simulink model "CCMLin2Mgr"  (R2022b)
settings: Solver=FixedStepDiscrete  StartTime=0.0  StopTime=10.0  FixedStep=0.02  RelTol=1e-3
undefined symbols (not defined in the file or in its folder): ComChlType, NetworkChlType, P_LinIfSchHandleType, P_Std_ReturnType
(part 2 of 3)

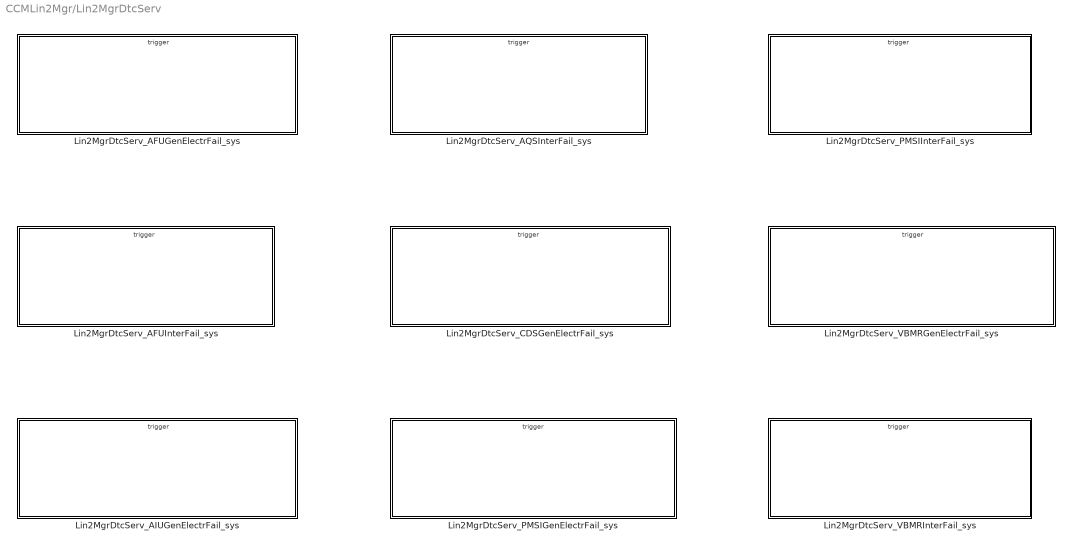
== system CCMLin2Mgr/Lin2MgrDtcServ ==
  Lin2MgrDtcServ_AFUGenElectrFail_sys : Subsystem
  Lin2MgrDtcServ_AFUInterFail_sys : Subsystem
  Lin2MgrDtcServ_AIUGenElectrFail_sys : Subsystem
  Lin2MgrDtcServ_AQSInterFail_sys : Subsystem
  Lin2MgrDtcServ_CDSGenElectrFail_sys : Subsystem
  Lin2MgrDtcServ_PMSIGenElectrFail_sys : Subsystem
  Lin2MgrDtcServ_PMSIInterFail_sys : Subsystem
  Lin2MgrDtcServ_VBMRGenElectrFail_sys : Subsystem
  Lin2MgrDtcServ_VBMRInterFail_sys : Subsystem

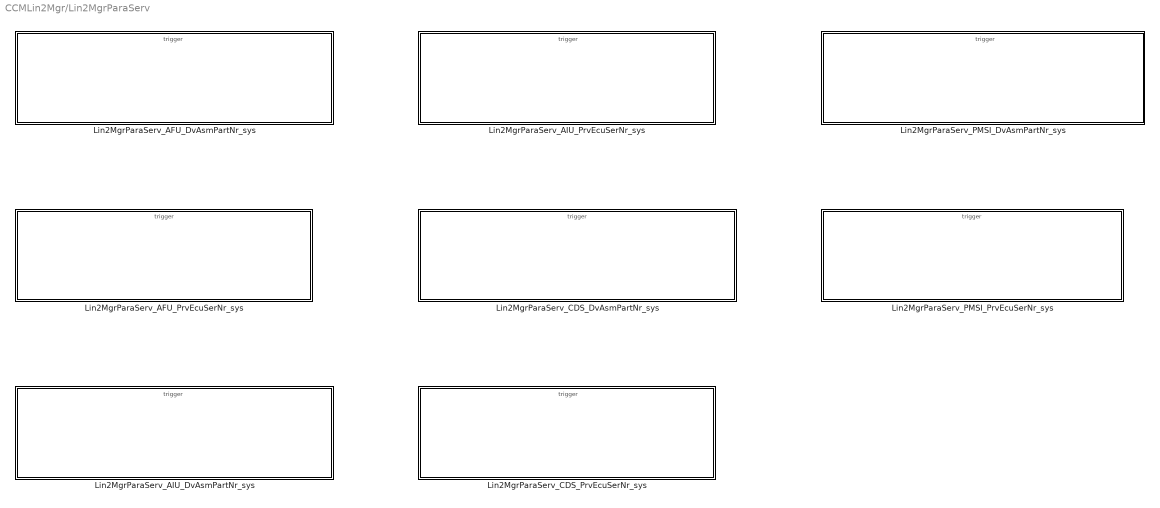
== system CCMLin2Mgr/Lin2MgrParaServ ==
  Lin2MgrParaServ_AFU_DvAsmPartNr_sys : Subsystem
  Lin2MgrParaServ_AFU_PrvEcuSerNr_sys : Subsystem
  Lin2MgrParaServ_AIU_DvAsmPartNr_sys : Subsystem
  Lin2MgrParaServ_AIU_PrvEcuSerNr_sys : Subsystem
  Lin2MgrParaServ_CDS_DvAsmPartNr_sys : Subsystem
  Lin2MgrParaServ_CDS_PrvEcuSerNr_sys : Subsystem
  Lin2MgrParaServ_PMSI_DvAsmPartNr_sys : Subsystem
  Lin2MgrParaServ_PMSI_PrvEcuSerNr_sys : Subsystem

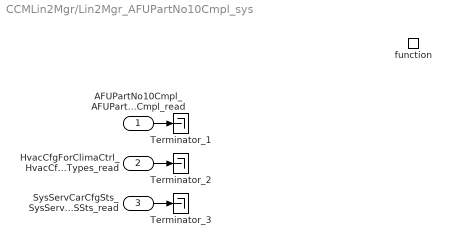
== system CCMLin2Mgr/Lin2Mgr_AFUPartNo10Cmpl_sys ==
  AFUPartNo10Cmpl_AFUPartNo10Cmpl_read : Inport
  HvacCfgForClimaCtrl_HvacCfgForAQSTypes_read : Inport  Port=2
  SysServCarCfgSts_SysServCCPAQSSts_read : Inport  Port=3
  function : TriggerPort  TriggerType=function-call  VariantControl=(inherit)
  Terminator_1 : Terminator
  Terminator_2 : Terminator
  Terminator_3 : Terminator
  wires:
  AFUPartNo10Cmpl_AFUPartNo10Cmpl_read/1 -> Terminator_1/1
  HvacCfgForClimaCtrl_HvacCfgForAQSTypes_read/1 -> Terminator_2/1
  SysServCarCfgSts_SysServCCPAQSSts_read/1 -> Terminator_3/1

== system CCMLin2Mgr/Lin2Mgr_AFUPartNoCmpl_sys ==
  AFUPartNoCmpl_AFUPartNoCmpl_read : Inport
  function : TriggerPort  TriggerType=function-call  VariantControl=(inherit)
  Terminator_1 : Terminator
  wires:
  AFUPartNoCmpl_AFUPartNoCmpl_read/1 -> Terminator_1/1

== system CCMLin2Mgr/Lin2Mgr_AFUSerNo_sys ==
  AFUSerNo_AFUSerNo_read : Inport
  function : TriggerPort  TriggerType=function-call  VariantControl=(inherit)
  Terminator_1 : Terminator
  wires:
  AFUSerNo_AFUSerNo_read/1 -> Terminator_1/1

== system CCMLin2Mgr/Lin2Mgr_AIUPartNo10Cmpl_sys ==
  AIUPartNo10Cmpl_AIUPartNo10Cmpl_read : Inport
  function : TriggerPort  TriggerType=function-call  VariantControl=(inherit)
  Terminator_1 : Terminator
  wires:
  AIUPartNo10Cmpl_AIUPartNo10Cmpl_read/1 -> Terminator_1/1

== system CCMLin2Mgr/Lin2Mgr_AIUPartNoCmpl_sys ==
  AIUPartNoCmpl_AIUPartNoCmpl_read : Inport
  function : TriggerPort  TriggerType=function-call  VariantControl=(inherit)
  Terminator_1 : Terminator
  wires:
  AIUPartNoCmpl_AIUPartNoCmpl_read/1 -> Terminator_1/1

== system CCMLin2Mgr/Lin2Mgr_AIUSerNo_sys ==
  AIUSerNo_AIUSerNo_read : Inport
  function : TriggerPort  TriggerType=function-call  VariantControl=(inherit)
  Terminator_1 : Terminator
  wires:
  AIUSerNo_AIUSerNo_read/1 -> Terminator_1/1

== system CCMLin2Mgr/Lin2Mgr_CDSPartNo10Cmpl_sys ==
  CDSPartNo10Cmpl_CDSPartNo10Cmpl_read : Inport
  function : TriggerPort  TriggerType=function-call  VariantControl=(inherit)
  Terminator_1 : Terminator
  wires:
  CDSPartNo10Cmpl_CDSPartNo10Cmpl_read/1 -> Terminator_1/1

== system CCMLin2Mgr/Lin2Mgr_CDSPartNoCmpl_sys ==
  CDSPartNoCmpl_CDSPartNoCmpl_read : Inport
  function : TriggerPort  TriggerType=function-call  VariantControl=(inherit)
  Terminator_1 : Terminator
  wires:
  CDSPartNoCmpl_CDSPartNoCmpl_read/1 -> Terminator_1/1

== system CCMLin2Mgr/Lin2Mgr_CDSSerNo_sys ==
  CDSSerNo_CDSSerNo_read : Inport
  function : TriggerPort  TriggerType=function-call  VariantControl=(inherit)
  Terminator_1 : Terminator
  wires:
  CDSSerNo_CDSSerNo_read/1 -> Terminator_1/1

== system CCMLin2Mgr/Lin2Mgr_Init ==
  Event Listener : EventListener  EventName=reset

== system CCMLin2Mgr/Lin2Mgr_KeepComActv_sys ==
  KeepComActvL2_KeepComActvL2_read : Inport
  function : TriggerPort  TriggerType=function-call  VariantControl=(inherit)  SampleTimeType=periodic  SampleTime=-1
  Terminator_1 : Terminator
  wires:
  KeepComActvL2_KeepComActvL2_read/1 -> Terminator_1/1

== system CCMLin2Mgr/Lin2Mgr_PMSIPartNo10Hw_sys ==
  PMSIPartNo10Hw_PMSIPartNo10Hw_read : Inport
  function : TriggerPort  TriggerType=function-call  VariantControl=(inherit)
  Terminator_1 : Terminator
  wires:
  PMSIPartNo10Hw_PMSIPartNo10Hw_read/1 -> Terminator_1/1

== system CCMLin2Mgr/Lin2Mgr_PMSIPartNoHw_sys ==
  PMSIPartNoHw_PMSIPartNoHw_read : Inport
  function : TriggerPort  TriggerType=function-call  VariantControl=(inherit)
  Terminator_1 : Terminator
  wires:
  PMSIPartNoHw_PMSIPartNoHw_read/1 -> Terminator_1/1

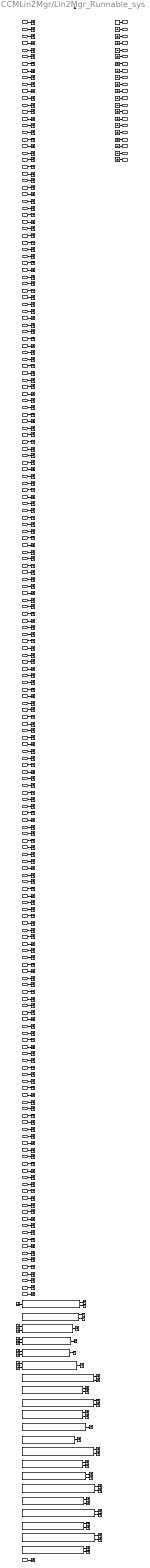
== system CCMLin2Mgr/Lin2Mgr_Runnable_sys ==
  RlyCmdCmft_RlyCmdCmft_read : Inport
  SysServCarCfgSts_SysServCarID_read : Inport  Port=2
  SysServCarCfgSts_SysServCCPAQSSts_read : Inport  Port=3
  SysServCarCfgSts_SysServCCPHEXSensorSts_read : Inport  Port=4
  SysServCarCfgSts_SysServCCPLin2AFUSts_read : Inport  Port=5
  SysServCarCfgSts_SysServCCPLin2AIUSts_read : Inport  Port=6
  SysServCarCfgSts_SysServCCPLin2CDSSts_read : Inport  Port=7
  SysServCarCfgSts_SysServCCPLin2PMSISts_read : Inport  Port=8
  SysServCarCfgSts_SysServCCPLin3VGASts_read : Inport  Port=9
  SysServCarCfgSts_SysServDUAirVentSnrSts_read : Inport  Port=10
  SysServCarCfgSts_SysServDUBlowerPwmSignSts_read : Inport  Port=11
  SysServCarCfgSts_SysServDUBlowerPwmSignTypeSts_read : Inport  Port=12
  SysServCarCfgSts_SysServDUBlowerPwmSts_read : Inport  Port=13
  SysServCarCfgSts_SysServDUDCDefrostSts_read : Inport  Port=14
  SysServCarCfgSts_SysServDUDCModeSts_read : Inport  Port=15
  SysServCarCfgSts_SysServDUDCRecSts_read : Inport  Port=16
  SysServCarCfgSts_SysServDUDCSModeSts_read : Inport  Port=17
  SysServCarCfgSts_SysServDUDCSTLSts_read : Inport  Port=18
  SysServCarCfgSts_SysServDUDCTLSts_read : Inport  Port=19
  SysServCarCfgSts_SysServDUDCTRSts_read : Inport  Port=20
  SysServCarCfgSts_SysServDUFLFootSts_read : Inport  Port=21
  SysServCarCfgSts_SysServDUFLVentSts_read : Inport  Port=22
  SysServCarCfgSts_SysServDUFRFootSts_read : Inport  Port=23
  SysServCarCfgSts_SysServDUFRVentSts_read : Inport  Port=24
  SysServCarCfgSts_SysServDUIncarMotorSts_read : Inport  Port=25
  SysServCarCfgSts_SysServDULin1HBMFSts_read : Inport  Port=26
  SysServCarCfgSts_SysServDULin4HFAFSts_read : Inport  Port=27
  SysServCarCfgSts_SysServDUSFLFootSts_read : Inport  Port=28
  SysServCarCfgSts_SysServDUSFLVentSts_read : Inport  Port=29
  SysServCarCfgSts_SysServDUSFRFootSts_read : Inport  Port=30
  SysServCarCfgSts_SysServDUSFRVentSts_read : Inport  Port=31
  SysServCarCfgSts_SysServDUSnsrForFCmptmtTSts_read : Inport  Port=32
  SysServCarCfgSts_SysServDUSnsrForRair_read : Inport  Port=33
  SysServCarCfgSts_SysServDUSnsrForRCmptmtTSts_read : Inport  Port=34
  AACAirClngErrSts_AACAirClngErrSts_read : Inport  Port=35
  AFUGenErr_AFUGenErr_read : Inport  Port=36
  AFUPartNo10Cmpl_AFUPartNo10Cmpl_read : Inport  Port=37
  AFUPartNoCmpl_AFUPartNoCmpl_read : Inport  Port=38
  AFUSerNo_AFUSerNo_read : Inport  Port=39
  AirClngErrSts_AirClngErrSts_read : Inport  Port=40
  AirFragFanErr_AirFragFanErr_read : Inport  Port=41
  AirFragMot1Err_AirFragMot1Err_read : Inport  Port=42
  AirFragMot2Err_AirFragMot2Err_read : Inport  Port=43
  AirFragMot3Err_AirFragMot3Err_read : Inport  Port=44
  AirFragMot4Err_AirFragMot4Err_read : Inport  Port=45
  AirFragMot5Err_AirFragMot5Err_read : Inport  Port=46
  AirFragOvrVoltErr_AirFragOvrVoltErr_read : Inport  Port=47
  AirFragTempErr_AirFragTempErr_read : Inport  Port=48
  AirFragUndVoltErr_AirFragUndVoltErr_read : Inport  Port=49
  AIUPartNo10Cmpl_AIUPartNo10Cmpl_read : Inport  Port=50
  AIUPartNoCmpl_AIUPartNoCmpl_read : Inport  Port=51
  AIUSerNo_AIUSerNo_read : Inport  Port=52
  AQSAirQuality_AQSAirQuality_read : Inport  Port=53
  AQSCOxstatus_AQSCOxstatus_read : Inport  Port=54
  AQSNH3status_AQSNH3status_read : Inport  Port=55
  AQSNoxStatus_AQSNoxStatus_read : Inport  Port=56
  CarCfgForClimaCtrl_CarCfgForAirClng_read : Inport  Port=57
  CarCfgForClimaCtrl_CarCfgForAirClnrAdv_read : Inport  Port=58
  CarCfgForClimaCtrl_CarCfgForAirFrag_read : Inport  Port=59
  CarCfgForClimaCtrl_CarCfgForAirMtrlSnsr_read : Inport  Port=60
  CarCfgForClimaCtrl_CarCfgForAirQly_read : Inport  Port=61
  CarCfgForClimaCtrl_CarCfgForAmntOfSeat_read : Inport  Port=62
  CarCfgForClimaCtrl_CarCfgForAutDemst_read : Inport  Port=63
  CarCfgForClimaCtrl_CarCfgForAuxWtrPmp_read : Inport  Port=64
  CarCfgForClimaCtrl_CarCfgForBRAND_read : Inport  Port=65
  CarCfgForClimaCtrl_CarCfgForBypVlvInHvac_read : Inport  Port=66
  CarCfgForClimaCtrl_CarCfgForCabinOxgenSupply_read : Inport  Port=67
  CarCfgForClimaCtrl_CarCfgForClsVlvInHvac_read : Inport  Port=68
  CarCfgForClimaCtrl_CarCfgForCmprAcTyp_read : Inport  Port=69
  CarCfgForClimaCtrl_CarCfgForCmptmtClng_read : Inport  Port=70
  CarCfgForClimaCtrl_CarCfgForElectricLiftingModule_read : Inport  Port=71
  CarCfgForClimaCtrl_CarCfgForElecVentAdjmt_read : Inport  Port=72
  CarCfgForClimaCtrl_CarCfgForExtVentnClimaCtrl_read : Inport  Port=73
  CarCfgForClimaCtrl_CarCfgForFlcTyp_read : Inport  Port=74
  CarCfgForClimaCtrl_CarCfgForFoldCSD_read : Inport  Port=75
  CarCfgForClimaCtrl_CarCfgForFuTyp_read : Inport  Port=76
  CarCfgForClimaCtrl_CarCfgForGlassTyp_read : Inport  Port=77
  CarCfgForClimaCtrl_CarCfgForHeadRadar_read : Inport  Port=78
  CarCfgForClimaCtrl_CarCfgForHeatPmp_read : Inport  Port=79
  CarCfgForClimaCtrl_CarCfgForHeatrDurgDrvg_read : Inport  Port=80
  CarCfgForClimaCtrl_CarCfgForHeatrTyp_read : Inport  Port=81
  CarCfgForClimaCtrl_CarCfgForHvacFilTyp_read : Inport  Port=82
  CarCfgForClimaCtrl_CarCfgForHvacFlrSnsrForT_read : Inport  Port=83
  CarCfgForClimaCtrl_CarCfgForHvacHeatrSnsrT_read : Inport  Port=84
  CarCfgForClimaCtrl_CarCfgForHvacRe_read : Inport  Port=85
  CarCfgForClimaCtrl_CarCfgForHvacTyp_read : Inport  Port=86
  CarCfgForClimaCtrl_CarCfgForInteriorViewCamera_read : Inport  Port=87
  CarCfgForClimaCtrl_CarCfgForLifeDetection_read : Inport  Port=88
  CarCfgForClimaCtrl_CarCfgForLoVoltElecCfg_read : Inport  Port=89
  CarCfgForClimaCtrl_CarCfgForNavSysandIntnmap_read : Inport  Port=90
  CarCfgForClimaCtrl_CarCfgForNrOfDoor_read : Inport  Port=91
  CarCfgForClimaCtrl_CarCfgForPipeInFuTank_read : Inport  Port=92
  CarCfgForClimaCtrl_CarCfgForPowerSeats_read : Inport  Port=93
  CarCfgForClimaCtrl_CarCfgForPreClng_read : Inport  Port=94
  CarCfgForClimaCtrl_CarCfgForPrkgCDSNotAvl_read : Inport  Port=95
  CarCfgForClimaCtrl_CarCfgForPrkgClima_read : Inport  Port=96
  CarCfgForClimaCtrl_CarCfgForPrpsnTyp_read : Inport  Port=97
  CarCfgForClimaCtrl_CarCfgForPTCInFrontHVAC_read : Inport  Port=98
  CarCfgForClimaCtrl_CarCfgForRemEngStrtPreCdn_read : Inport  Port=99
  CarCfgForClimaCtrl_CarCfgForRoofHatch_read : Inport  Port=100
  CarCfgForClimaCtrl_CarCfgForSeatHeatgCal_read : Inport  Port=101
  CarCfgForClimaCtrl_CarCfgForSeatHeatgForRowFirst_read : Inport  Port=102
  CarCfgForClimaCtrl_CarCfgForSeatHeatgForRowSec_read : Inport  Port=103
  CarCfgForClimaCtrl_CarCfgForSeatVentnForRowFirst_read : Inport  Port=104
  CarCfgForClimaCtrl_CarCfgForSeatVentnForRowSec_read : Inport  Port=105
  CarCfgForClimaCtrl_CarCfgForSecRowVentnCenter_read : Inport  Port=106
  CarCfgForClimaCtrl_CarCfgForSecRowVentnSide_read : Inport  Port=107
  CarCfgForClimaCtrl_CarCfgForSmallFlowCyc_read : Inport  Port=108
  CarCfgForClimaCtrl_CarCfgForSmkSnsr_read : Inport  Port=109
  CarCfgForClimaCtrl_CarCfgForSnsrForCmptmtT_read : Inport  Port=110
  CarCfgForClimaCtrl_CarCfgForSnsrHumPrsnt_read : Inport  Port=111
  CarCfgForClimaCtrl_CarCfgForStartDevice_read : Inport  Port=112
  CarCfgForClimaCtrl_CarCfgForSteerWhlHeatg_read : Inport  Port=113
  CarCfgForClimaCtrl_CarCfgForSteerWhlPosn_read : Inport  Port=114
  CarCfgForClimaCtrl_CarCfgForSunSnsr_read : Inport  Port=115
  CarCfgForClimaCtrl_CarCfgForTelmNodTyp_read : Inport  Port=116
  CarCfgForClimaCtrl_CarCfgForThermMngt_read : Inport  Port=117
  CarCfgForClimaCtrl_CarCfgForVehTyp_read : Inport  Port=118
  CarCfgForClimaCtrl_CarCfgForVentLiftMotorExiSts_read : Inport  Port=119
  CarCfgForClimaCtrl_CarCfgForVgaMotorExistSts_read : Inport  Port=120
  CarCfgForClimaCtrl_CarCfgForWadingAssistSystem_read : Inport  Port=121
  CarCfgForClimaCtrl_CarCfgForWindHeatd_read : Inport  Port=122
  CarCfgForClimaCtrl_CarCfgWindFollowHumanMov_read : Inport  Port=123
  CDSPartNo10Cmpl_CDSPartNo10Cmpl_read : Inport  Port=124
  CDSPartNoCmpl_CDSPartNoCmpl_read : Inport  Port=125
  CDSSerNo_CDSSerNo_read : Inport  Port=126
  ClimRlyCmd_ClimRlyCmd_read : Inport  Port=127
  CmpmtInAirPmAQI_CmpmtInAirPmAQI_read : Inport  Port=128
  CmpmtInAirPmDnsty_CmpmtInAirPmDnsty_read : Inport  Port=129
  CmptmtOxygenConcQFNgcc_CmptmtOxygenConcQFNgcc_read : Inport  Port=130
  CmptmtOxygenConcVluNgcc_CmptmtOxygenConcVluNgcc_read : Inport  Port=131
  HvacCfgForClimaCtrl_HvacCfgForAQSTypes_read : Inport  Port=132
  HvacCfgForClimaCtrl_HvacCfgForHvacFrontBlowerType_read : Inport  Port=133
  HvacCfgForClimaCtrl_HvacCfgForHvacLowSideRETXVESts_read : Inport  Port=134
  HvacCfgForClimaCtrl_HvacCfgForHvacMiscExistSts_read : Inport  Port=135
  HvacCfgForClimaCtrl_HvacCfgForHvacMotorExistSts_read : Inport  Port=136
  HvacCfgForClimaCtrl_HvacCfgForHvacReBlowerType_read : Inport  Port=137
  HvacCfgForClimaCtrl_HvacCfgForHvacReVentPstn_read : Inport  Port=138
  HvacCfgForClimaCtrl_HvacCfgForHvacSensorExistSts_read : Inport  Port=139
  HvacCfgForClimaCtrl_HvacCfgForHvacSnsrRCmptmtESts_read : Inport  Port=140
  HvacCfgForClimaCtrl_HvacCfgForHvacSnsrReHexESts_read : Inport  Port=141
  HvacFanReqRe_HvacFanReqRe_read : Inport  Port=142
  HvacFanSts2HvacFanBattU2_HvacFanSts2HvacFanBattU2_read : Inport  Port=143
  HvacFanSts2HvacFanExt2_HvacFanSts2HvacFanExt2_read : Inport  Port=144
  HvacFanSts2HvacFanHw2_HvacFanSts2HvacFanHw2_read : Inport  Port=145
  HvacFanSts2HvacFanMotU2_HvacFanSts2HvacFanMotU2_read : Inport  Port=146
  HvacFanSts2HvacFanReSts1_HvacFanSts2HvacFanReSts1_read : Inport  Port=147
  HvacFanSts2HvacFanReSts2_HvacFanSts2HvacFanReSts2_read : Inport  Port=148
  HvacFanSts2HvacFanSw2_HvacFanSts2HvacFanSw2_read : Inport  Port=149
  HvacFanSts2HvacFanT2_HvacFanSts2HvacFanT2_read : Inport  Port=150
  HvacFanVoltReqRe_HvacFanVoltReqRe_read : Inport  Port=151
  InPm25FanErr_InPm25FanErr_read : Inport  Port=152
  InPm25SensrIntErr_InPm25SensrIntErr_read : Inport  Port=153
  InPm25SnrTempErr_InPm25SnrTempErr_read : Inport  Port=154
  InPm25SnrVoltgRangErr_InPm25SnrVoltgRangErr_read : Inport  Port=155
  InPm25SnsrElecErr_InPm25SnsrElecErr_read : Inport  Port=156
  InPm25SnsrSts_InPm25SnsrSts_read : Inport  Port=157
  IntCo2CircElecErr_IntCo2CircElecErr_read : Inport  Port=158
  IntCo2HvErr_IntCo2HvErr_read : Inport  Port=159
  IntCo2LvErr_IntCo2LvErr_read : Inport  Port=160
  KeepComActvL2_KeepComActvL2_read : Inport  Port=161
  OxygenConcSnsrElecErr_OxygenConcSnsrElecErr_read : Inport  Port=162
  OxygenConcSnsrElecErrNgcc_OxygenConcSnsrElecErrNgcc_read : Inport  Port=163
  OxygenConcSnsrIntErr_OxygenConcSnsrIntErr_read : Inport  Port=164
  OxygenConcSnsrIntErrNgcc_OxygenConcSnsrIntErrNgcc_read : Inport  Port=165
  OxygenConcSnsrOvrVoltRangErr_OxygenConcSnsrOvrVoltRangErr_read : Inport  Port=166
  OxygenConcSnsrStsNgcc_OxygenConcSnsrStsNgcc_read : Inport  Port=167
  OxygenConcSnsrTempErr_OxygenConcSnsrTempErr_read : Inport  Port=168
  OxygenConcSnsrTempErrNgcc_OxygenConcSnsrTempErrNgcc_read : Inport  Port=169
  OxygenConcSnsrUndVoltRangErr_OxygenConcSnsrUndVoltRangErr_read : Inport  Port=170
  OxygenConcSnsrUndVoltRangErrNgcc_OxygenConcSnsrUndVoltRangErrNgcc_read : Inport  Port=171
  PMSIPartNo10Hw_PMSIPartNo10Hw_read : Inport  Port=172
  PMSIPartNoHw_PMSIPartNoHw_read : Inport  Port=173
  PwrSplyToSnsrForAirQly_PwrSplyToSnsrForAirQly_read : Inport  Port=174
  RHFAScheTableReq_Val_read : Inport  Port=175
  SerNoPMSI_SerNoPMSI_read : Inport  Port=176
  SysServCarCfgSts_SysServCCPBGMNum_read : Inport  Port=177
  SysServCarCfgSts_SysServCCPLin2AQSSts_read : Inport  Port=178
  SysServCarCfgSts_SysServDUDCFLMdeSts_read : Inport  Port=179
  SysServCarCfgSts_SysServDUReBlowerPwmSignSts_read : Inport  Port=180
  SysServCarCfgSts_SysServDUReBlowerPwmSts_read : Inport  Port=181
  SysServCarCfgSts_SysServFrntHEXSnsrPosn_read : Inport  Port=182
  SysServCarCfgSts_SysServLowSideRETXVSts_read : Inport  Port=183
  SysServCarCfgSts_SysServReHEXSnsrPosn_read : Inport  Port=184
  SysServCarCfgSts_SysServRHFASts_read : Inport  Port=185
  SysServCarCfgSts_SysServSnsrForFCmptmtTType_read : Inport  Port=186
  BswLifeCycle_LifeCycle_read : Inport  Port=187
  function : TriggerPort  TriggerType=function-call  VariantControl=(inherit)  SampleTimeType=periodic  SampleTime=-1
  Ground_{1..9,11..30} (29 alike) : Ground
  Ground_10 : Subsystem
  LinIfServ_GetScheduleInfo : FunctionCaller  FunctionPrototype=[ScheduleInfo,ERR] = LinIfServ_GetScheduleInfo(Channel)  InputArgumentSpecifications=NetworkChlType(0)  OutputArgumentSpecifications=P_LinIfScheduleInfoType, P_Std_ReturnType
  LinSMServ_ScheduleRequest : FunctionCaller  FunctionPrototype=ERR = LinSMServ_ScheduleRequest(network,schedule)  InputArgumentSpecifications=NetworkChlType(0), P_LinIfSchHandleType  OutputArgumentSpecifications=P_Std_ReturnType
  LinService_GetLin2WakeupStatus : FunctionCaller  FunctionPrototype=[WakSta,ERR] = LinService_GetLin2WakeupStatus()  OutputArgumentSpecifications=uint8(1), P_Std_ReturnType
  LinService_SendLin3dFrame : FunctionCaller  FunctionPrototype=ERR = LinService_SendLin3dFrame(channel,data)  InputArgumentSpecifications=ComChlType(0), uint8([1])  OutputArgumentSpecifications=P_Std_ReturnType
  LinService_SetLin3cFrame : FunctionCaller  FunctionPrototype=ERR = LinService_SetLin3cFrame(Channel,Data)  InputArgumentSpecifications=ComChlType(0), uint8([1])  OutputArgumentSpecifications=P_Std_ReturnType
  LinService_SetLinTable : FunctionCaller  FunctionPrototype=ERR = LinService_SetLinTable(Channel,Table)  InputArgumentSpecifications=ComChlType(0), uint8(1)  OutputArgumentSpecifications=P_Std_ReturnType
  MirrorLin2ErrServ_GetAQSCcmLin2Fr01NoResp : FunctionCaller  FunctionPrototype=ErrFlag = MirrorLin2ErrServ_GetAQSCcmLin2Fr01NoResp()  OutputArgumentSpecifications=uint8(1)
  MirrorLin2ErrServ_GetAQSRxErrSta : FunctionCaller  FunctionPrototype=ErrFlag = MirrorLin2ErrServ_GetAQSRxErrSta()  OutputArgumentSpecifications=uint8(1)
  MirrorLin2ErrServ_GetAfuCcmLin2Fr01NoResp : FunctionCaller  FunctionPrototype=[ErrFlag,ERR] = MirrorLin2ErrServ_GetAfuCcmLin2Fr01NoResp()  OutputArgumentSpecifications=uint8(1), P_Std_ReturnType
  MirrorLin2ErrServ_GetAfuRxErrSta : FunctionCaller  FunctionPrototype=[ErrFlag,ERR] = MirrorLin2ErrServ_GetAfuRxErrSta()  OutputArgumentSpecifications=uint8(1), P_Std_ReturnType
  MirrorLin2ErrServ_GetAiuCcmLin2Fr01NoResp : FunctionCaller  FunctionPrototype=[ErrFlag,ERR] = MirrorLin2ErrServ_GetAiuCcmLin2Fr01NoResp()  OutputArgumentSpecifications=uint8(1), P_Std_ReturnType
  MirrorLin2ErrServ_GetAiuRxErrSta : FunctionCaller  FunctionPrototype=[ErrFlag,ERR] = MirrorLin2ErrServ_GetAiuRxErrSta()  OutputArgumentSpecifications=uint8(1), P_Std_ReturnType
  MirrorLin2ErrServ_GetCdsCcmLin2Fr01NoResp : FunctionCaller  FunctionPrototype=[ErrFlag,ERR] = MirrorLin2ErrServ_GetCdsCcmLin2Fr01NoResp()  OutputArgumentSpecifications=uint8(1), P_Std_ReturnType
  MirrorLin2ErrServ_GetCdsRxErrSta : FunctionCaller  FunctionPrototype=[ErrFlag,ERR] = MirrorLin2ErrServ_GetCdsRxErrSta()  OutputArgumentSpecifications=uint8(1), P_Std_ReturnType
  MirrorLin2ErrServ_GetLinBusTxErrSta : FunctionCaller  FunctionPrototype=[ErrFlag,ERR] = MirrorLin2ErrServ_GetLinBusTxErrSta()  OutputArgumentSpecifications=uint8(1), P_Std_ReturnType
  MirrorLin2ErrServ_GetPmsiCcmLin2Fr01NoResp : FunctionCaller  FunctionPrototype=[ErrFlag,ERR] = MirrorLin2ErrServ_GetPmsiCcmLin2Fr01NoResp()  OutputArgumentSpecifications=uint8(1), P_Std_ReturnType
  MirrorLin2ErrServ_GetPmsiRxErrSta : FunctionCaller  FunctionPrototype=[ErrFlag,ERR] = MirrorLin2ErrServ_GetPmsiRxErrSta()  OutputArgumentSpecifications=uint8(1), P_Std_ReturnType
  MirrorLin2ErrServ_GetRhfaCcmLin2Fr01NoResp : FunctionCaller  FunctionPrototype=[ErrFlag,ERR] = MirrorLin2ErrServ_GetRhfaCcmLin2Fr01NoResp()  OutputArgumentSpecifications=uint8(1), P_Std_ReturnType
  MirrorLin2ErrServ_GetRhfaRxErrSta : FunctionCaller  FunctionPrototype=[ErrFlag,ERR] = MirrorLin2ErrServ_GetRhfaRxErrSta()  OutputArgumentSpecifications=uint8(1), P_Std_ReturnType
  MirrorLin2ErrServ_GetVbmrCcmLin2Fr01NoResp : FunctionCaller  FunctionPrototype=[ErrFlag,ERR] = MirrorLin2ErrServ_GetVbmrCcmLin2Fr01NoResp()  OutputArgumentSpecifications=uint8(1), P_Std_ReturnType
  MirrorLin2ErrServ_GetVbmrRxErrSta : FunctionCaller  FunctionPrototype=[ErrFlag,ERR] = MirrorLin2ErrServ_GetVbmrRxErrSta()  OutputArgumentSpecifications=uint8(1), P_Std_ReturnType
  Terminator_{1..223} (223 alike) : Terminator
  AirQlyLin_AirQlyLin_write : Outport
      settings: EnsureOutportIsVirtual=on
  HvacFanSet2HvacFanRxFrq2_HvacFanSet2HvacFanRxFrq2_write : Outport  Port=2
      settings: EnsureOutportIsVirtual=on
  Lin2ActvReady_Lin2ActvReady_write : Outport  Port=3
      settings: EnsureOutportIsVirtual=on
  Lin2MgrDtcServ2_AACGenElectrFail_write : Outport  Port=4
      settings: EnsureOutportIsVirtual=on
  Lin2MgrDtcServ2_IOSMGenElectrFail_write : Outport  Port=5
      settings: EnsureOutportIsVirtual=on
  Lin2MgrDtcServ2_IOSMInterFail_write : Outport  Port=6
      settings: EnsureOutportIsVirtual=on
  ReVbmrSts_ReVbmrSts_write : Outport  Port=7
      settings: EnsureOutportIsVirtual=on
  AirFragErrSts_AirFragErrSts_write : Outport  Port=8
      settings: EnsureOutportIsVirtual=on
  AirPmQf_AirPmQf_write : Outport  Port=9
      settings: EnsureOutportIsVirtual=on
  HvacFanSet2HvacFanIMaxMax2_HvacFanSet2HvacFanIMaxMax2_write : Outport  Port=10
      settings: EnsureOutportIsVirtual=on
  HvacFanSet2HvacFanIMaxMin2_HvacFanSet2HvacFanIMaxMin2_write : Outport  Port=11
      settings: EnsureOutportIsVirtual=on
  HvacFanSet2HvacFanKneeU2_HvacFanSet2HvacFanKneeU2_write : Outport  Port=12
      settings: EnsureOutportIsVirtual=on
  HvacFanSet2HvacFanRamp2_HvacFanSet2HvacFanRamp2_write : Outport  Port=13
      settings: EnsureOutportIsVirtual=on
  HvacFanSet2HvacFanSeln2_HvacFanSet2HvacFanSeln2_write : Outport  Port=14
      settings: EnsureOutportIsVirtual=on
  HvacFanSet2HvacFanSftyRunThd2_HvacFanSet2HvacFanSftyRunThd2_write : Outport  Port=15
      settings: EnsureOutportIsVirtual=on
  HvacFanSet2HvacFanSpd2_HvacFanSet2HvacFanSpd2_write : Outport  Port=16
      settings: EnsureOutportIsVirtual=on
  HvacFanSet2HvacSftyRunSpd2_HvacFanSet2HvacSftyRunSpd2_write : Outport  Port=17
      settings: EnsureOutportIsVirtual=on
  IntPm25LvlFrmClima_IntPm25LvlFrmClima_write : Outport  Port=18
      settings: EnsureOutportIsVirtual=on
  IntPm25StsFrmClima_IntPm25StsFrmClima_write : Outport  Port=19
      settings: EnsureOutportIsVirtual=on
  IntPm25VluFrmClima_IntPm25VluFrmClima_write : Outport  Port=20
      settings: EnsureOutportIsVirtual=on
  LIN2ScheTableReq_Val_write : Outport  Port=21
      settings: EnsureOutportIsVirtual=on
  wires:
  RlyCmdCmft_RlyCmdCmft_read/1 -> Terminator_1/1
  SysServCarCfgSts_SysServCarID_read/1 -> Terminator_2/1
  SysServCarCfgSts_SysServCCPAQSSts_read/1 -> Terminator_3/1
  SysServCarCfgSts_SysServCCPHEXSensorSts_read/1 -> Terminator_4/1
  SysServCarCfgSts_SysServCCPLin2AFUSts_read/1 -> Terminator_5/1
  SysServCarCfgSts_SysServCCPLin2AIUSts_read/1 -> Terminator_6/1
  SysServCarCfgSts_SysServCCPLin2CDSSts_read/1 -> Terminator_7/1
  SysServCarCfgSts_SysServCCPLin2PMSISts_read/1 -> Terminator_8/1
  SysServCarCfgSts_SysServCCPLin3VGASts_read/1 -> Terminator_9/1
  SysServCarCfgSts_SysServDUAirVentSnrSts_read/1 -> Terminator_10/1
  SysServCarCfgSts_SysServDUBlowerPwmSignSts_read/1 -> Terminator_11/1
  SysServCarCfgSts_SysServDUBlowerPwmSignTypeSts_read/1 -> Terminator_12/1
  SysServCarCfgSts_SysServDUBlowerPwmSts_read/1 -> Terminator_13/1
  SysServCarCfgSts_SysServDUDCDefrostSts_read/1 -> Terminator_14/1
  SysServCarCfgSts_SysServDUDCModeSts_read/1 -> Terminator_15/1
  SysServCarCfgSts_SysServDUDCRecSts_read/1 -> Terminator_16/1
  SysServCarCfgSts_SysServDUDCSModeSts_read/1 -> Terminator_17/1